simulink model "CCMMotor"  (R2022b)
settings: Solver=FixedStepDiscrete  StartTime=0.0  StopTime=10.0  FixedStep=0.01  RelTol=1e-3
undefined symbols (not defined in the file or in its folder): DcChlType, HFAChlType, MtrRoutStaEnum, P_Std_ReturnType, VGAChlType
(part 2 of 4)

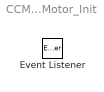
== system CCMMotor/CCMMotor_Init ==
  Event Listener : EventListener  EventName=reset
